simulink model "CCMSysServ"  (R2022b)
settings: Solver=FixedStepDiscrete  StartTime=0.0  StopTime=10.0  FixedStep=0.05  RelTol=1e-3
model workspace (MAT data in the model file):
  NvMBlockCarCfgData : Simulink.Signal (value not decoded)
  NvMBlockCarCfgDataInit : Simulink.Parameter (value not decoded)
  NvMBlockDidDataD005 : Simulink.Signal (value not decoded)
  NvMBlockDidDataD005Init : Simulink.Parameter (value not decoded)
  NvMBlockDidDataD09A : Simulink.Signal (value not decoded)
  NvMBlockDidDataD09AInit : Simulink.Parameter (value not decoded)
  NvMBlockDidDataE103 : Simulink.Signal (value not decoded)
  NvMBlockDidDataE103Init : Simulink.Parameter (value not decoded)
  NvMBlockDidDataF190 : Simulink.Signal (value not decoded)
  NvMBlockDidDataF190Init : Simulink.Parameter (value not decoded)
  NvMBlockDidDataF1AB : Simulink.Signal (value not decoded)
  NvMBlockDidDataF1ABInit : Simulink.Parameter (value not decoded)
symbols that are objects in the model workspace (value not decoded): NvMBlockCarCfgDataInit, NvMBlockDidDataD005Init, NvMBlockDidDataD09AInit, NvMBlockDidDataE103Init, NvMBlockDidDataF190Init, NvMBlockDidDataF1ABInit
(part 4 of 4)

== system CCMSysServ/CCMSysServ_VehCfgPrmExt_sys ==
  VehCfgPrmExt_VehCfgPrmExt_read : Inport
  function : TriggerPort  TriggerType=function-call  VariantControl=(inherit)
  PA_NvMBlockCarCfgDataInit : Constant  Value=NvMBlockCarCfgDataInit
  PA_NvMBlockDidDataD005Init : Constant  Value=NvMBlockDidDataD005Init
  PA_NvMBlockDidDataD09AInit : Constant  Value=NvMBlockDidDataD09AInit
  PA_NvMBlockDidDataE103Init : Constant  Value=NvMBlockDidDataE103Init
  PA_NvMBlockDidDataF190Init : Constant  Value=NvMBlockDidDataF190Init
  PA_NvMBlockDidDataF1ABInit : Constant  Value=NvMBlockDidDataF1ABInit
  Terminator_{1..7} (7 alike) : Terminator
  wires:
  VehCfgPrmExt_VehCfgPrmExt_read/1 -> Terminator_1/1
  PA_NvMBlockCarCfgDataInit/1 -> Terminator_2/1
  PA_NvMBlockDidDataD005Init/1 -> Terminator_3/1
  PA_NvMBlockDidDataD09AInit/1 -> Terminator_4/1
  PA_NvMBlockDidDataE103Init/1 -> Terminator_5/1
  PA_NvMBlockDidDataF190Init/1 -> Terminator_6/1
  PA_NvMBlockDidDataF1ABInit/1 -> Terminator_7/1

== system CCMSysServ/CCMSysServ_VehCfgPrm_sys ==
  VehCfgPrm_VehCfgPrm_read : Inport
  function : TriggerPort  TriggerType=function-call  VariantControl=(inherit)
  PA_NvMBlockCarCfgDataInit : Constant  Value=NvMBlockCarCfgDataInit
  PA_NvMBlockDidDataD005Init : Constant  Value=NvMBlockDidDataD005Init
  PA_NvMBlockDidDataD09AInit : Constant  Value=NvMBlockDidDataD09AInit
  PA_NvMBlockDidDataE103Init : Constant  Value=NvMBlockDidDataE103Init
  PA_NvMBlockDidDataF190Init : Constant  Value=NvMBlockDidDataF190Init
  PA_NvMBlockDidDataF1ABInit : Constant  Value=NvMBlockDidDataF1ABInit
  Terminator_{1..7} (7 alike) : Terminator
  wires:
  VehCfgPrm_VehCfgPrm_read/1 -> Terminator_1/1
  PA_NvMBlockCarCfgDataInit/1 -> Terminator_2/1
  PA_NvMBlockDidDataD005Init/1 -> Terminator_3/1
  PA_NvMBlockDidDataD09AInit/1 -> Terminator_4/1
  PA_NvMBlockDidDataE103Init/1 -> Terminator_5/1
  PA_NvMBlockDidDataF190Init/1 -> Terminator_6/1
  PA_NvMBlockDidDataF1ABInit/1 -> Terminator_7/1

== system CCMSysServ/CCMSysServ_VehModMngtGlbSafe1_sys ==
  VehModMngtGlbSafe1_VehModMngtGlbSafe1_read : Inport
  function : TriggerPort  TriggerType=function-call  VariantControl=(inherit)
  PA_NvMBlockCarCfgDataInit : Constant  Value=NvMBlockCarCfgDataInit
  PA_NvMBlockDidDataD005Init : Constant  Value=NvMBlockDidDataD005Init
  PA_NvMBlockDidDataD09AInit : Constant  Value=NvMBlockDidDataD09AInit
  PA_NvMBlockDidDataE103Init : Constant  Value=NvMBlockDidDataE103Init
  PA_NvMBlockDidDataF190Init : Constant  Value=NvMBlockDidDataF190Init
  PA_NvMBlockDidDataF1ABInit : Constant  Value=NvMBlockDidDataF1ABInit
  Terminator_{1..7} (7 alike) : Terminator
  wires:
  VehModMngtGlbSafe1_VehModMngtGlbSafe1_read/1 -> Terminator_1/1
  PA_NvMBlockCarCfgDataInit/1 -> Terminator_2/1
  PA_NvMBlockDidDataD005Init/1 -> Terminator_3/1
  PA_NvMBlockDidDataD09AInit/1 -> Terminator_4/1
  PA_NvMBlockDidDataE103Init/1 -> Terminator_5/1
  PA_NvMBlockDidDataF190Init/1 -> Terminator_6/1
  PA_NvMBlockDidDataF1ABInit/1 -> Terminator_7/1

== system CCMSysServ/CCMSysServ_Vin_sys ==
  Vin_Vin_read : Inport
  function : TriggerPort  TriggerType=function-call  VariantControl=(inherit)
  PA_NvMBlockCarCfgDataInit : Constant  Value=NvMBlockCarCfgDataInit
  PA_NvMBlockDidDataD005Init : Constant  Value=NvMBlockDidDataD005Init
  PA_NvMBlockDidDataD09AInit : Constant  Value=NvMBlockDidDataD09AInit
  PA_NvMBlockDidDataE103Init : Constant  Value=NvMBlockDidDataE103Init
  PA_NvMBlockDidDataF190Init : Constant  Value=NvMBlockDidDataF190Init
  PA_NvMBlockDidDataF1ABInit : Constant  Value=NvMBlockDidDataF1ABInit
  Terminator_{1..7} (7 alike) : Terminator
  wires:
  Vin_Vin_read/1 -> Terminator_1/1
  PA_NvMBlockCarCfgDataInit/1 -> Terminator_2/1
  PA_NvMBlockDidDataD005Init/1 -> Terminator_3/1
  PA_NvMBlockDidDataD09AInit/1 -> Terminator_4/1
  PA_NvMBlockDidDataE103Init/1 -> Terminator_5/1
  PA_NvMBlockDidDataF190Init/1 -> Terminator_6/1
  PA_NvMBlockDidDataF1ABInit/1 -> Terminator_7/1

== system CCMSysServ/SysServDtcService ==
  SysServDtcService_GetCCPInvalidConfig_sys : Subsystem
  SysServDtcService_GetCCPInvalidSwc_sys : Subsystem
  SysServDtcService_GetCCPNoConfigState_sys : Subsystem
  SysServDtcService_GetFltEgyCnsWdSts_ToFaltFlag_sys : Subsystem
  SysServDtcService_GetVinNotProgIncmp_sys : Subsystem

== system CCMSysServ/SysServParaService ==
  SysServParaService_ReadCarConfigParaFaultCnt_sys : Subsystem
  SysServParaService_ReadData_DIDD005_sys : Subsystem
  SysServParaService_ReadData_DIDD09A_sys : Subsystem
  SysServParaService_ReadData_DIDF190_sys : Subsystem
  SysServParaService_ReadData_DIDF1AB_sys : Subsystem
  SysServParaService_WriteData_DIDD09A_sys : Subsystem
  SysServParaService_WriteData_DIDF1AB_sys : Subsystem

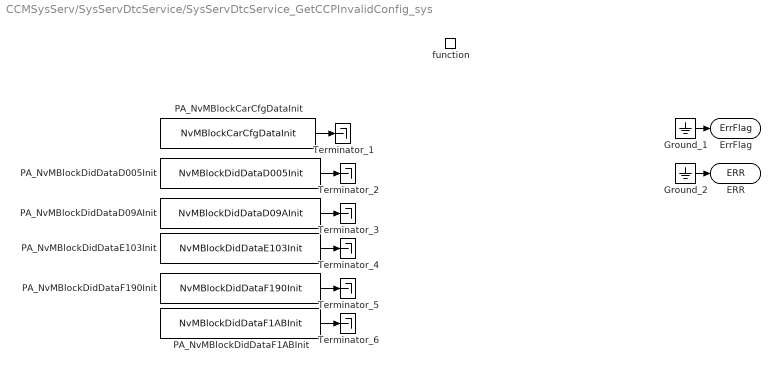
== system CCMSysServ/SysServDtcService/SysServDtcService_GetCCPInvalidConfig_sys ==
  function : TriggerPort  TriggerType=function-call  IsSimulinkFunction=on  FunctionName=SysServDtcService_GetCCPInvalidConfig  VariantControl=(inherit)
  Ground_1 : Ground
  Ground_2 : Ground
  PA_NvMBlockCarCfgDataInit : Constant  Value=NvMBlockCarCfgDataInit
  PA_NvMBlockDidDataD005Init : Constant  Value=NvMBlockDidDataD005Init
  PA_NvMBlockDidDataD09AInit : Constant  Value=NvMBlockDidDataD09AInit
  PA_NvMBlockDidDataE103Init : Constant  Value=NvMBlockDidDataE103Init
  PA_NvMBlockDidDataF190Init : Constant  Value=NvMBlockDidDataF190Init
  PA_NvMBlockDidDataF1ABInit : Constant  Value=NvMBlockDidDataF1ABInit
  Terminator_{1..6} (6 alike) : Terminator
  ErrFlag : ArgOut  OutDataTypeStr=Enum: DTCState  ArgumentName=ErrFlag
  ERR : ArgOut  Port=2  OutDataTypeStr=Std_ReturnType  ArgumentName=ERR
  wires:
  PA_NvMBlockCarCfgDataInit/1 -> Terminator_1/1
  PA_NvMBlockDidDataD005Init/1 -> Terminator_2/1
  PA_NvMBlockDidDataD09AInit/1 -> Terminator_3/1
  PA_NvMBlockDidDataE103Init/1 -> Terminator_4/1
  PA_NvMBlockDidDataF190Init/1 -> Terminator_5/1
  PA_NvMBlockDidDataF1ABInit/1 -> Terminator_6/1
  Ground_1/1 -> ErrFlag/1
  Ground_2/1 -> ERR/1

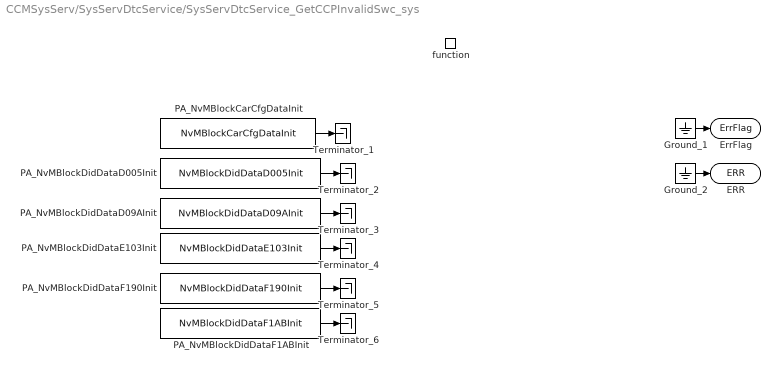
== system CCMSysServ/SysServDtcService/SysServDtcService_GetCCPInvalidSwc_sys ==
  function : TriggerPort  TriggerType=function-call  IsSimulinkFunction=on  FunctionName=SysServDtcService_GetCCPInvalidSwc  VariantControl=(inherit)
  Ground_1 : Ground
  Ground_2 : Ground
  PA_NvMBlockCarCfgDataInit : Constant  Value=NvMBlockCarCfgDataInit
  PA_NvMBlockDidDataD005Init : Constant  Value=NvMBlockDidDataD005Init
  PA_NvMBlockDidDataD09AInit : Constant  Value=NvMBlockDidDataD09AInit
  PA_NvMBlockDidDataE103Init : Constant  Value=NvMBlockDidDataE103Init
  PA_NvMBlockDidDataF190Init : Constant  Value=NvMBlockDidDataF190Init
  PA_NvMBlockDidDataF1ABInit : Constant  Value=NvMBlockDidDataF1ABInit
  Terminator_{1..6} (6 alike) : Terminator
  ErrFlag : ArgOut  OutDataTypeStr=Enum: DTCState  ArgumentName=ErrFlag
  ERR : ArgOut  Port=2  OutDataTypeStr=Std_ReturnType  ArgumentName=ERR
  wires:
  PA_NvMBlockCarCfgDataInit/1 -> Terminator_1/1
  PA_NvMBlockDidDataD005Init/1 -> Terminator_2/1
  PA_NvMBlockDidDataD09AInit/1 -> Terminator_3/1
  PA_NvMBlockDidDataE103Init/1 -> Terminator_4/1
  PA_NvMBlockDidDataF190Init/1 -> Terminator_5/1
  PA_NvMBlockDidDataF1ABInit/1 -> Terminator_6/1
  Ground_1/1 -> ErrFlag/1
  Ground_2/1 -> ERR/1

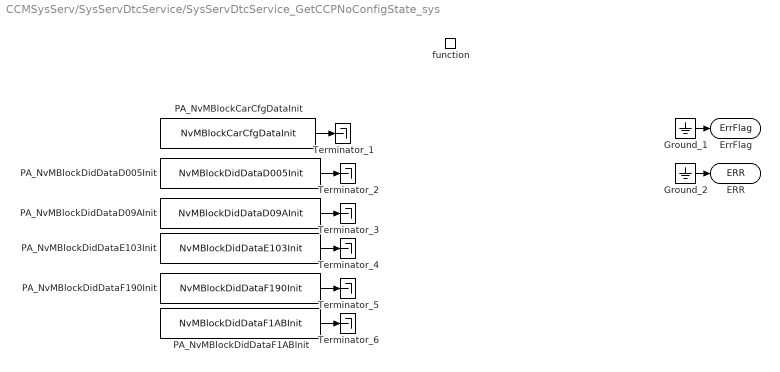
== system CCMSysServ/SysServDtcService/SysServDtcService_GetCCPNoConfigState_sys ==
  function : TriggerPort  TriggerType=function-call  IsSimulinkFunction=on  FunctionName=SysServDtcService_GetCCPNoConfigState  VariantControl=(inherit)
  Ground_1 : Ground
  Ground_2 : Ground
  PA_NvMBlockCarCfgDataInit : Constant  Value=NvMBlockCarCfgDataInit
  PA_NvMBlockDidDataD005Init : Constant  Value=NvMBlockDidDataD005Init
  PA_NvMBlockDidDataD09AInit : Constant  Value=NvMBlockDidDataD09AInit
  PA_NvMBlockDidDataE103Init : Constant  Value=NvMBlockDidDataE103Init
  PA_NvMBlockDidDataF190Init : Constant  Value=NvMBlockDidDataF190Init
  PA_NvMBlockDidDataF1ABInit : Constant  Value=NvMBlockDidDataF1ABInit
  Terminator_{1..6} (6 alike) : Terminator
  ErrFlag : ArgOut  OutDataTypeStr=Enum: DTCState  ArgumentName=ErrFlag
  ERR : ArgOut  Port=2  OutDataTypeStr=Std_ReturnType  ArgumentName=ERR
  wires:
  PA_NvMBlockCarCfgDataInit/1 -> Terminator_1/1
  PA_NvMBlockDidDataD005Init/1 -> Terminator_2/1
  PA_NvMBlockDidDataD09AInit/1 -> Terminator_3/1
  PA_NvMBlockDidDataE103Init/1 -> Terminator_4/1
  PA_NvMBlockDidDataF190Init/1 -> Terminator_5/1
  PA_NvMBlockDidDataF1ABInit/1 -> Terminator_6/1
  Ground_1/1 -> ErrFlag/1
  Ground_2/1 -> ERR/1

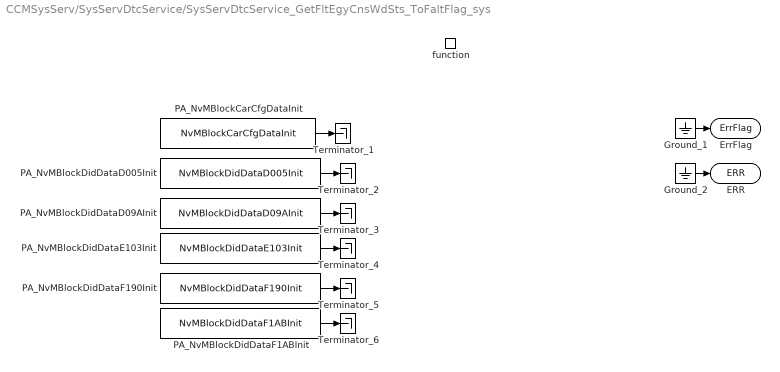
== system CCMSysServ/SysServDtcService/SysServDtcService_GetFltEgyCnsWdSts_ToFaltFlag_sys ==
  function : TriggerPort  TriggerType=function-call  IsSimulinkFunction=on  FunctionName=SysServDtcService_GetFltEgyCnsWdSts_ToFaltFlag  VariantControl=(inherit)
  Ground_1 : Ground
  Ground_2 : Ground
  PA_NvMBlockCarCfgDataInit : Constant  Value=NvMBlockCarCfgDataInit
  PA_NvMBlockDidDataD005Init : Constant  Value=NvMBlockDidDataD005Init
  PA_NvMBlockDidDataD09AInit : Constant  Value=NvMBlockDidDataD09AInit
  PA_NvMBlockDidDataE103Init : Constant  Value=NvMBlockDidDataE103Init
  PA_NvMBlockDidDataF190Init : Constant  Value=NvMBlockDidDataF190Init
  PA_NvMBlockDidDataF1ABInit : Constant  Value=NvMBlockDidDataF1ABInit
  Terminator_{1..6} (6 alike) : Terminator
  ErrFlag : ArgOut  OutDataTypeStr=Enum: DTCState  ArgumentName=ErrFlag
  ERR : ArgOut  Port=2  OutDataTypeStr=Std_ReturnType  ArgumentName=ERR
  wires:
  PA_NvMBlockCarCfgDataInit/1 -> Terminator_1/1
  PA_NvMBlockDidDataD005Init/1 -> Terminator_2/1
  PA_NvMBlockDidDataD09AInit/1 -> Terminator_3/1
  PA_NvMBlockDidDataE103Init/1 -> Terminator_4/1
  PA_NvMBlockDidDataF190Init/1 -> Terminator_5/1
  PA_NvMBlockDidDataF1ABInit/1 -> Terminator_6/1
  Ground_1/1 -> ErrFlag/1
  Ground_2/1 -> ERR/1

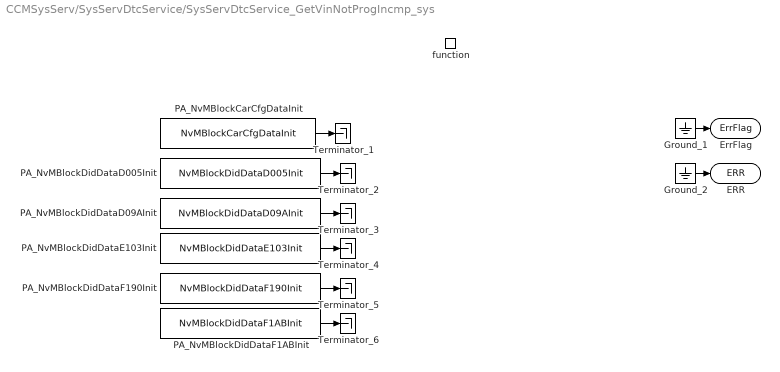
== system CCMSysServ/SysServDtcService/SysServDtcService_GetVinNotProgIncmp_sys ==
  function : TriggerPort  TriggerType=function-call  IsSimulinkFunction=on  FunctionName=SysServDtcService_GetVinNotProgIncmp  VariantControl=(inherit)
  Ground_1 : Ground
  Ground_2 : Ground
  PA_NvMBlockCarCfgDataInit : Constant  Value=NvMBlockCarCfgDataInit
  PA_NvMBlockDidDataD005Init : Constant  Value=NvMBlockDidDataD005Init
  PA_NvMBlockDidDataD09AInit : Constant  Value=NvMBlockDidDataD09AInit
  PA_NvMBlockDidDataE103Init : Constant  Value=NvMBlockDidDataE103Init
  PA_NvMBlockDidDataF190Init : Constant  Value=NvMBlockDidDataF190Init
  PA_NvMBlockDidDataF1ABInit : Constant  Value=NvMBlockDidDataF1ABInit
  Terminator_{1..6} (6 alike) : Terminator
  ErrFlag : ArgOut  OutDataTypeStr=Enum: DTCState  ArgumentName=ErrFlag
  ERR : ArgOut  Port=2  OutDataTypeStr=Std_ReturnType  ArgumentName=ERR
  wires:
  PA_NvMBlockCarCfgDataInit/1 -> Terminator_1/1
  PA_NvMBlockDidDataD005Init/1 -> Terminator_2/1
  PA_NvMBlockDidDataD09AInit/1 -> Terminator_3/1
  PA_NvMBlockDidDataE103Init/1 -> Terminator_4/1
  PA_NvMBlockDidDataF190Init/1 -> Terminator_5/1
  PA_NvMBlockDidDataF1ABInit/1 -> Terminator_6/1
  Ground_1/1 -> ErrFlag/1
  Ground_2/1 -> ERR/1

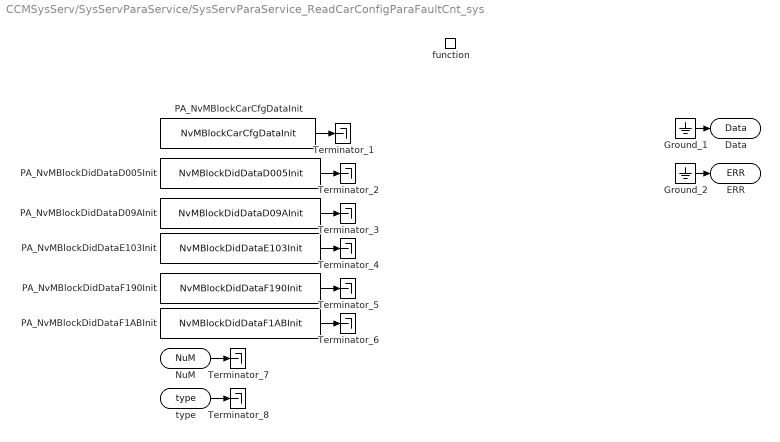
== system CCMSysServ/SysServParaService/SysServParaService_ReadCarConfigParaFaultCnt_sys ==
  function : TriggerPort  TriggerType=function-call  IsSimulinkFunction=on  FunctionName=SysServParaService_ReadCarConfigParaFaultCnt  VariantControl=(inherit)
  Ground_1 : Ground
  Ground_2 : Ground
  PA_NvMBlockCarCfgDataInit : Constant  Value=NvMBlockCarCfgDataInit
  PA_NvMBlockDidDataD005Init : Constant  Value=NvMBlockDidDataD005Init
  PA_NvMBlockDidDataD09AInit : Constant  Value=NvMBlockDidDataD09AInit
  PA_NvMBlockDidDataE103Init : Constant  Value=NvMBlockDidDataE103Init
  PA_NvMBlockDidDataF190Init : Constant  Value=NvMBlockDidDataF190Init
  PA_NvMBlockDidDataF1ABInit : Constant  Value=NvMBlockDidDataF1ABInit
  Terminator_{1..8} (8 alike) : Terminator
  NuM : ArgIn  OutDataTypeStr=UInt8  ArgumentName=NuM
  type : ArgIn  Port=2  OutDataTypeStr=Enum: CCPFaultType  ArgumentName=type
  Data : ArgOut  OutDataTypeStr=UInt8  PortDimensions=2  ArgumentName=Data
  ERR : ArgOut  Port=2  OutDataTypeStr=Std_ReturnType  ArgumentName=ERR
  wires:
  PA_NvMBlockCarCfgDataInit/1 -> Terminator_1/1
  PA_NvMBlockDidDataD005Init/1 -> Terminator_2/1
  PA_NvMBlockDidDataD09AInit/1 -> Terminator_3/1
  PA_NvMBlockDidDataE103Init/1 -> Terminator_4/1
  PA_NvMBlockDidDataF190Init/1 -> Terminator_5/1
  PA_NvMBlockDidDataF1ABInit/1 -> Terminator_6/1
  NuM/1 -> Terminator_7/1
  type/1 -> Terminator_8/1
  Ground_1/1 -> Data/1
  Ground_2/1 -> ERR/1

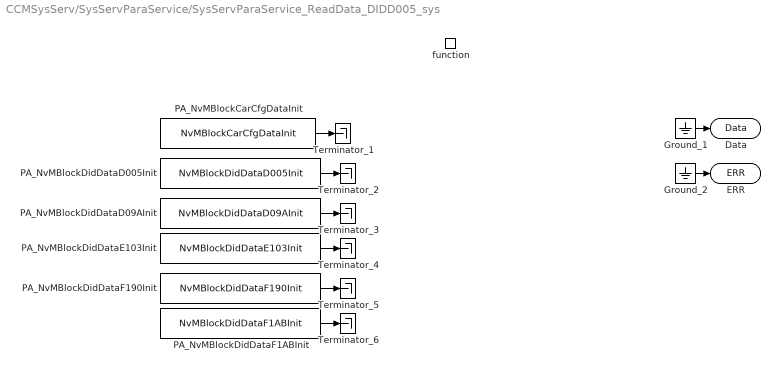
== system CCMSysServ/SysServParaService/SysServParaService_ReadData_DIDD005_sys ==
  function : TriggerPort  TriggerType=function-call  IsSimulinkFunction=on  FunctionName=SysServParaService_ReadData_DIDD005  VariantControl=(inherit)
  Ground_1 : Ground
  Ground_2 : Ground
  PA_NvMBlockCarCfgDataInit : Constant  Value=NvMBlockCarCfgDataInit
  PA_NvMBlockDidDataD005Init : Constant  Value=NvMBlockDidDataD005Init
  PA_NvMBlockDidDataD09AInit : Constant  Value=NvMBlockDidDataD09AInit
  PA_NvMBlockDidDataE103Init : Constant  Value=NvMBlockDidDataE103Init
  PA_NvMBlockDidDataF190Init : Constant  Value=NvMBlockDidDataF190Init
  PA_NvMBlockDidDataF1ABInit : Constant  Value=NvMBlockDidDataF1ABInit
  Terminator_{1..6} (6 alike) : Terminator
  Data : ArgOut  OutDataTypeStr=UInt8  PortDimensions=90  ArgumentName=Data
  ERR : ArgOut  Port=2  OutDataTypeStr=Std_ReturnType  ArgumentName=ERR
  wires:
  PA_NvMBlockCarCfgDataInit/1 -> Terminator_1/1
  PA_NvMBlockDidDataD005Init/1 -> Terminator_2/1
  PA_NvMBlockDidDataD09AInit/1 -> Terminator_3/1
  PA_NvMBlockDidDataE103Init/1 -> Terminator_4/1
  PA_NvMBlockDidDataF190Init/1 -> Terminator_5/1
  PA_NvMBlockDidDataF1ABInit/1 -> Terminator_6/1
  Ground_1/1 -> Data/1
  Ground_2/1 -> ERR/1

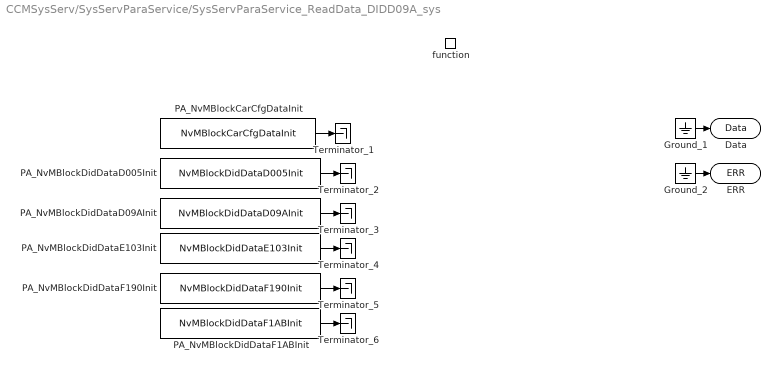
== system CCMSysServ/SysServParaService/SysServParaService_ReadData_DIDD09A_sys ==
  function : TriggerPort  TriggerType=function-call  IsSimulinkFunction=on  FunctionName=SysServParaService_ReadData_DIDD09A  VariantControl=(inherit)
  Ground_1 : Ground
  Ground_2 : Ground
  PA_NvMBlockCarCfgDataInit : Constant  Value=NvMBlockCarCfgDataInit
  PA_NvMBlockDidDataD005Init : Constant  Value=NvMBlockDidDataD005Init
  PA_NvMBlockDidDataD09AInit : Constant  Value=NvMBlockDidDataD09AInit
  PA_NvMBlockDidDataE103Init : Constant  Value=NvMBlockDidDataE103Init
  PA_NvMBlockDidDataF190Init : Constant  Value=NvMBlockDidDataF190Init
  PA_NvMBlockDidDataF1ABInit : Constant  Value=NvMBlockDidDataF1ABInit
  Terminator_{1..6} (6 alike) : Terminator
  Data : ArgOut  OutDataTypeStr=UInt8  ArgumentName=Data
  ERR : ArgOut  Port=2  OutDataTypeStr=Std_ReturnType  ArgumentName=ERR
  wires:
  PA_NvMBlockCarCfgDataInit/1 -> Terminator_1/1
  PA_NvMBlockDidDataD005Init/1 -> Terminator_2/1
  PA_NvMBlockDidDataD09AInit/1 -> Terminator_3/1
  PA_NvMBlockDidDataE103Init/1 -> Terminator_4/1
  PA_NvMBlockDidDataF190Init/1 -> Terminator_5/1
  PA_NvMBlockDidDataF1ABInit/1 -> Terminator_6/1
  Ground_1/1 -> Data/1
  Ground_2/1 -> ERR/1

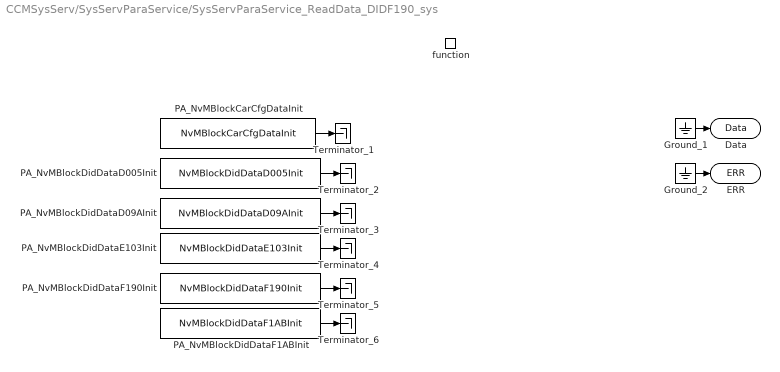
== system CCMSysServ/SysServParaService/SysServParaService_ReadData_DIDF190_sys ==
  function : TriggerPort  TriggerType=function-call  IsSimulinkFunction=on  FunctionName=SysServParaService_ReadData_DIDF190  VariantControl=(inherit)
  Ground_1 : Ground
  Ground_2 : Ground
  PA_NvMBlockCarCfgDataInit : Constant  Value=NvMBlockCarCfgDataInit
  PA_NvMBlockDidDataD005Init : Constant  Value=NvMBlockDidDataD005Init
  PA_NvMBlockDidDataD09AInit : Constant  Value=NvMBlockDidDataD09AInit
  PA_NvMBlockDidDataE103Init : Constant  Value=NvMBlockDidDataE103Init
  PA_NvMBlockDidDataF190Init : Constant  Value=NvMBlockDidDataF190Init
  PA_NvMBlockDidDataF1ABInit : Constant  Value=NvMBlockDidDataF1ABInit
  Terminator_{1..6} (6 alike) : Terminator
  Data : ArgOut  OutDataTypeStr=UInt8  PortDimensions=16  ArgumentName=Data
  ERR : ArgOut  Port=2  OutDataTypeStr=Std_ReturnType  ArgumentName=ERR
  wires:
  PA_NvMBlockCarCfgDataInit/1 -> Terminator_1/1
  PA_NvMBlockDidDataD005Init/1 -> Terminator_2/1
  PA_NvMBlockDidDataD09AInit/1 -> Terminator_3/1
  PA_NvMBlockDidDataE103Init/1 -> Terminator_4/1
  PA_NvMBlockDidDataF190Init/1 -> Terminator_5/1
  PA_NvMBlockDidDataF1ABInit/1 -> Terminator_6/1
  Ground_1/1 -> Data/1
  Ground_2/1 -> ERR/1

== system CCMSysServ/SysServParaService/SysServParaService_ReadData_DIDF1AB_sys ==
  function : TriggerPort  TriggerType=function-call  IsSimulinkFunction=on  FunctionName=SysServParaService_ReadData_DIDF1AB  VariantControl=(inherit)
  Ground_1 : Ground
  Ground_2 : Ground
  PA_NvMBlockCarCfgDataInit : Constant  Value=NvMBlockCarCfgDataInit
  PA_NvMBlockDidDataD005Init : Constant  Value=NvMBlockDidDataD005Init
  PA_NvMBlockDidDataD09AInit : Constant  Value=NvMBlockDidDataD09AInit
  PA_NvMBlockDidDataE103Init : Constant  Value=NvMBlockDidDataE103Init
  PA_NvMBlockDidDataF190Init : Constant  Value=NvMBlockDidDataF190Init
  PA_NvMBlockDidDataF1ABInit : Constant  Value=NvMBlockDidDataF1ABInit
  Terminator_{1..6} (6 alike) : Terminator
  Data : ArgOut  OutDataTypeStr=UInt8  PortDimensions=8  ArgumentName=Data
  ERR : ArgOut  Port=2  OutDataTypeStr=Std_ReturnType  ArgumentName=ERR
  wires:
  PA_NvMBlockCarCfgDataInit/1 -> Terminator_1/1
  PA_NvMBlockDidDataD005Init/1 -> Terminator_2/1
  PA_NvMBlockDidDataD09AInit/1 -> Terminator_3/1
  PA_NvMBlockDidDataE103Init/1 -> Terminator_4/1
  PA_NvMBlockDidDataF190Init/1 -> Terminator_5/1
  PA_NvMBlockDidDataF1ABInit/1 -> Terminator_6/1
  Ground_1/1 -> Data/1
  Ground_2/1 -> ERR/1

== system CCMSysServ/SysServParaService/SysServParaService_WriteData_DIDD09A_sys ==
  function : TriggerPort  TriggerType=function-call  IsSimulinkFunction=on  FunctionName=SysServParaService_WriteData_DIDD09A  VariantControl=(inherit)
  Ground_1 : Ground
  PA_NvMBlockCarCfgDataInit : Constant  Value=NvMBlockCarCfgDataInit
  PA_NvMBlockDidDataD005Init : Constant  Value=NvMBlockDidDataD005Init
  PA_NvMBlockDidDataD09AInit : Constant  Value=NvMBlockDidDataD09AInit
  PA_NvMBlockDidDataE103Init : Constant  Value=NvMBlockDidDataE103Init
  PA_NvMBlockDidDataF190Init : Constant  Value=NvMBlockDidDataF190Init
  PA_NvMBlockDidDataF1ABInit : Constant  Value=NvMBlockDidDataF1ABInit
  Terminator_{1..7} (7 alike) : Terminator
  Data : ArgIn  OutDataTypeStr=UInt8  ArgumentName=Data
  ERR : ArgOut  OutDataTypeStr=Std_ReturnType  ArgumentName=ERR
  wires:
  PA_NvMBlockCarCfgDataInit/1 -> Terminator_1/1
  PA_NvMBlockDidDataD005Init/1 -> Terminator_2/1
  PA_NvMBlockDidDataD09AInit/1 -> Terminator_3/1
  PA_NvMBlockDidDataE103Init/1 -> Terminator_4/1
  PA_NvMBlockDidDataF190Init/1 -> Terminator_5/1
  PA_NvMBlockDidDataF1ABInit/1 -> Terminator_6/1
  Data/1 -> Terminator_7/1
  Ground_1/1 -> ERR/1

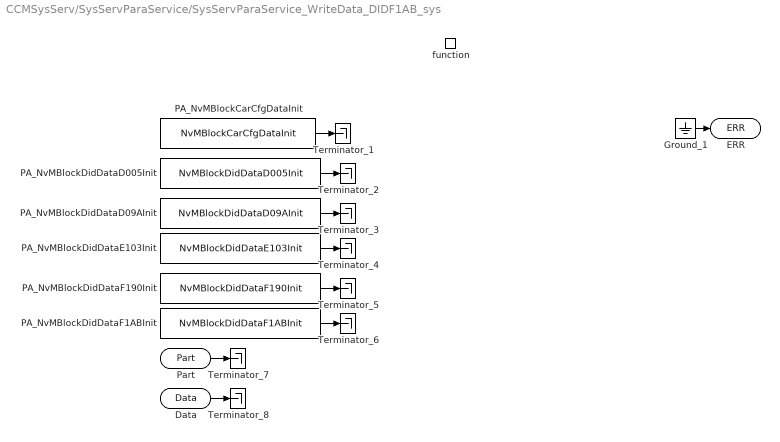
== system CCMSysServ/SysServParaService/SysServParaService_WriteData_DIDF1AB_sys ==
  function : TriggerPort  TriggerType=function-call  IsSimulinkFunction=on  FunctionName=SysServParaService_WriteData_DIDF1AB  VariantControl=(inherit)
  Ground_1 : Ground
  PA_NvMBlockCarCfgDataInit : Constant  Value=NvMBlockCarCfgDataInit
  PA_NvMBlockDidDataD005Init : Constant  Value=NvMBlockDidDataD005Init
  PA_NvMBlockDidDataD09AInit : Constant  Value=NvMBlockDidDataD09AInit
  PA_NvMBlockDidDataE103Init : Constant  Value=NvMBlockDidDataE103Init
  PA_NvMBlockDidDataF190Init : Constant  Value=NvMBlockDidDataF190Init
  PA_NvMBlockDidDataF1ABInit : Constant  Value=NvMBlockDidDataF1ABInit
  Terminator_{1..8} (8 alike) : Terminator
  Part : ArgIn  OutDataTypeStr=SignalPartType  ArgumentName=Part
  Data : ArgIn  Port=2  OutDataTypeStr=UInt8  PortDimensions=8  ArgumentName=Data
  ERR : ArgOut  OutDataTypeStr=Std_ReturnType  ArgumentName=ERR
  wires:
  PA_NvMBlockCarCfgDataInit/1 -> Terminator_1/1
  PA_NvMBlockDidDataD005Init/1 -> Terminator_2/1
  PA_NvMBlockDidDataD09AInit/1 -> Terminator_3/1
  PA_NvMBlockDidDataE103Init/1 -> Terminator_4/1
  PA_NvMBlockDidDataF190Init/1 -> Terminator_5/1
  PA_NvMBlockDidDataF1ABInit/1 -> Terminator_6/1
  Part/1 -> Terminator_7/1
  Data/1 -> Terminator_8/1
  Ground_1/1 -> ERR/1
